simulink model "CtrlrCmptmtT"  (R2022b)
settings: Solver=FixedStepDiscrete  StartTime=0.0  StopTime=10.0  FixedStep=1  RelTol=1e-3
settings Model: Creator=blue.ge  LastModifiedBy=blue.ge
settings DataIOCC: SaveFormat=StructureWithTime
settings OptimizationCC: DefaultParameterBehavior=Inlined  EnhancedBackFolding=on  PassReuseOutputArgsAs=Individual arguments  ZeroExternalMemoryAtStartup=off  ZeroInternalMemoryAtStartup=off  OptimizeBlockOrder=speed  DifferentSizesBufferReuse=on  OptimizationCustomize=off  EfficientTunableParamExpr=on
settings DebuggingCC: ParameterTunabilityLossMsg=error
settings ModelReferenceCC: ModelReferenceNumInstancesAllowed=Single
settings SFSimCC: MATLABDynamicMemAlloc=off
settings RTWCC: Description=AUTOSAR  SystemTargetFile=autosar.tlc  GenCodeOnly=on  TemplateMakefile=ert_default_tmf
settings CodeAppCC: ForceParamTrailComments=on  IgnoreCustomStorageClasses=off  OperatorAnnotations=on  SimulinkDataObjDesc=on  SFDataObjDesc=on  InsertBlockDesc=on
settings STFCustomTargetCC: TargetLangStandard=C99 (ISO)  MultiwordLength=256  ParMdlRefBuildCompliant=on  CompOptLevelCompliant=on  IncludeMdlTerminateFcn=off  CombineOutputUpdateFcns=on  SuppressErrorStatus=on  ERTCustomFileBanners=on  SupportNonFinite=off  SupportComplex=off  SupportContinuousTime=off  SupportNonInlinedSFcns=off  RemoveResetFunc=on  MATLABClassNameForMDSCustomization=Simulink.SoftwareTarget.ERTCustomization  AutosarCompliant=on  UseToolchainInfoCompliant=on  LookupTableObjectStructAxisOrder=2,1,3,4,...  LUTObjectStructOrderEvenSpacing=Size,Table,Breakpoints  SystemTargetFile=autosar.tlc
settings ERTTargetCC: TargetLangStandard=C99 (ISO)  ConcurrentExecutionCompliant=off  IncludeMdlTerminateFcn=off  SuppressErrorStatus=on  SupportNonFinite=off  SupportComplex=off  ModelStepFunctionPrototypeControlCompliant=off  CPPClassGenCompliant=off  AutosarCompliant=on  LookupTableObjectStructAxisOrder=2,1,3,4,...  LUTObjectStructOrderEvenSpacing=Size,Table,Breakpoints  InlinedParameterPlacement=NonHierarchical  ERTCustomFileTemplate=example_file_process.tlc

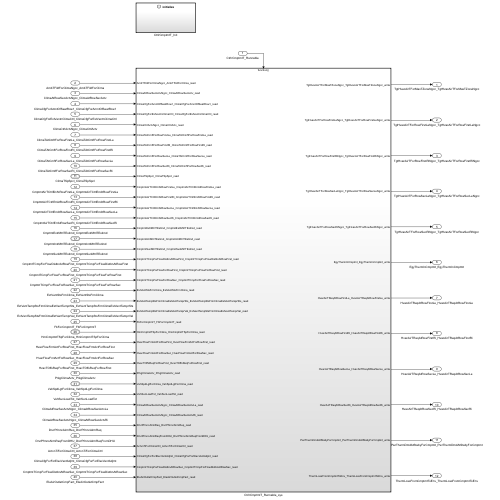
[thumbnail: Simulink's stored preview of the top-level diagram]
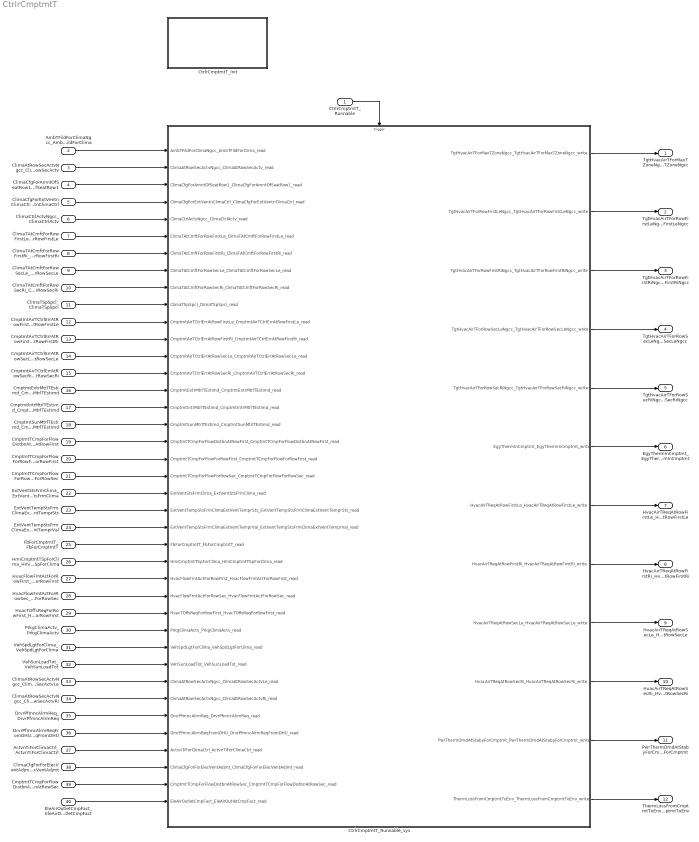
== system CtrlrCmptmtT ==
  CtrlrCmptmtT_Runnable : Inport  SampleTime=1  OutputFunctionCall=on
  AmbTFildForClimaNgcc_AmbTFildForClima : Inport  Port=2  OutDataTypeStr=T30  PortDimensions=1
  ClimaAtRowSecActvNgcc_ClimaAtRowSecActv : Inport  Port=3  OutDataTypeStr=Boolean  PortDimensions=1
  ClimaCfgForAmntOfSeatRow1_ClimaCfgForAmntOfSeatRow1 : Inport  Port=4  OutDataTypeStr=Enum: ClimaCfgForAmntOfSeatRow1  PortDimensions=1
  ClimaCfgForExtVentnClimaCtrl_ClimaCfgForExtVentnClimaCtrl : Inport  Port=5  OutDataTypeStr=Enum: CarCfgForExtVentnClimaCtrl  PortDimensions=1
  ClimaCtrlActvNgcc_ClimaCtrlActv : Inport  Port=6  OutDataTypeStr=Boolean  PortDimensions=1
  ClimaTAtCmftForRowFirstLe_ClimaTAtCmftForRowFirstLe : Inport  Port=7  OutDataTypeStr=T30  PortDimensions=1
  ClimaTAtCmftForRowFirstRi_ClimaTAtCmftForRowFirstRi : Inport  Port=8  OutDataTypeStr=T30  PortDimensions=1
  ClimaTAtCmftForRowSecLe_ClimaTAtCmftForRowSecLe : Inport  Port=9  OutDataTypeStr=T30  PortDimensions=1
  ClimaTAtCmftForRowSecRi_ClimaTAtCmftForRowSecRi : Inport  Port=10  OutDataTypeStr=T30  PortDimensions=1
  ClimaTSpSpcl_ClimaTSpSpcl : Inport  Port=11  OutDataTypeStr=Bus: ClimaTSpSpcl  PortDimensions=1  BusOutputAsStruct=on
  CmptmtAirTCtrlErrAtRowFirstLe_CmptmtAirTCtrlErrAtRowFirstLe : Inport  Port=12  OutDataTypeStr=T30  PortDimensions=1
  CmptmtAirTCtrlErrAtRowFirstRi_CmptmtAirTCtrlErrAtRowFirstRi : Inport  Port=13  OutDataTypeStr=T30  PortDimensions=1
  CmptmtAirTCtrlErrAtRowSecLe_CmptmtAirTCtrlErrAtRowSecLe : Inport  Port=14  OutDataTypeStr=T30  PortDimensions=1
  CmptmtAirTCtrlErrAtRowSecRi_CmptmtAirTCtrlErrAtRowSecRi : Inport  Port=15  OutDataTypeStr=T30  PortDimensions=1
  CmptmtExtrMtrlTEstimd_CmptmtExtrMtrlTEstimd : Inport  Port=16  OutDataTypeStr=T30  PortDimensions=1
  CmptmtIntrMtrlTEstimd_CmptmtIntrMtrlTEstimd : Inport  Port=17  OutDataTypeStr=Bus: MtrlSnsrT  PortDimensions=1  BusOutputAsStruct=on
  CmptmtSunMtrlTEstimd_CmptmtSunMtrlTEstimd : Inport  Port=18  OutDataTypeStr=T30  PortDimensions=1
  CmptmtTCmpForFlowDistbnAtRowFirst_CmptmtTCmpForFlowDistbnAtRowFirst : Inport  Port=19  OutDataTypeStr=Fac30  PortDimensions=1
  CmptmtTCmpForFlowForRowFirst_CmptmtTCmpForFlowForRowFirst : Inport  Port=20  OutDataTypeStr=Fac30  PortDimensions=1
  CmptmtTCmpForFlowForRowSec_CmptmtTCmpForFlowForRowSec : Inport  Port=21  OutDataTypeStr=Fac30  PortDimensions=1
  ExtVentStsFrmClima_ExtVentStsFrmClima : Inport  Port=22  OutDataTypeStr=Enum: OnOff1  PortDimensions=1
  ExtVentTempStsFrmClimaExtVentTemprSts_ExtVentTempStsFrmClimaExtVentTemprSts : Inport  Port=23  OutDataTypeStr=Enum: ExtVentTemprSts  PortDimensions=1
  ExtVentTempStsFrmClimaExtVentTemprVal_ExtVentTempStsFrmClimaExtVentTemprVal : Inport  Port=24  OutDataTypeStr=Temp5  PortDimensions=1
  FbForCmptmtT_FbForCmptmtT : Inport  Port=25  OutDataTypeStr=T30  PortDimensions=1
  HmiCmptmtTSpForClima_HmiCmptmtTSpForClima : Inport  Port=26  OutDataTypeStr=Bus: HmiCmptmtTSp  PortDimensions=1  BusOutputAsStruct=on
  HvacFlowFrntActForRowFirst_HvacFlowFrntActForRowFirst : Inport  Port=27  OutDataTypeStr=AirFlow30  PortDimensions=1
  HvacFlowFrntActForRowSec_HvacFlowFrntActForRowSec : Inport  Port=28  OutDataTypeStr=AirFlow30  PortDimensions=1
  HvacTOffsReqForRowFirst_HvacTOffsReqForRowFirst : Inport  Port=29  OutDataTypeStr=T30  PortDimensions=1
  PrkgClimaActv_PrkgClimaActv : Inport  Port=30  OutDataTypeStr=Enum: PrkgClimaActv  PortDimensions=1
  VehSpdLgtForClima_VehSpdLgtForClima : Inport  Port=31  OutDataTypeStr=Bus: VehSpdLgtForClima  PortDimensions=1  BusOutputAsStruct=on
  VehSunLoadTot_VehSunLoadTot : Inport  Port=32  OutDataTypeStr=SunLoad30  PortDimensions=1
  ClimaAtRowSecActvNgcc_ClimaAtRowSecActvLe : Inport  Port=33  OutDataTypeStr=Boolean  PortDimensions=1
  ClimaAtRowSecActvNgcc_ClimaAtRowSecActvRi : Inport  Port=34  OutDataTypeStr=Boolean  PortDimensions=1
  DrvrPfmncAlrmReq_DrvrPfmncAlrmReq : Inport  Port=35  OutDataTypeStr=Enum: DrvrPfmncWarnReq  PortDimensions=1
  DrvrPfmncAlrmReqFromDHU_DrvrPfmncAlrmReqFromDHU : Inport  Port=36  OutDataTypeStr=Enum: DrvrPfmncWarnReq  PortDimensions=1
  ActvnTiForClimaCtrl_ActvnTiForClimaCtrl : Inport  Port=37  OutDataTypeStr=Ti33  PortDimensions=1
  ClimaCfgForForElecVentAdjmt_ClimaCfgForForElecVentAdjmt : Inport  Port=38  OutDataTypeStr=Boolean  PortDimensions=1
  CmptmtTCmpForFlowDistbnAtRowSec_CmptmtTCmpForFlowDistbnAtRowSec : Inport  Port=39  OutDataTypeStr=Fac30  PortDimensions=1
  EleAirOutletCmpFact_EleAirOutletCmpFact : Inport  Port=40  OutDataTypeStr=VCCFac30  PortDimensions=1
  CtrlrCmptmtT_Init : Subsystem
  CtrlrCmptmtT_Runnable_sys : Subsystem
  TgtHvacAirTForMaxTZoneNgcc_TgtHvacAirTForMaxTZoneNgcc : Outport  OutDataTypeStr=T30  PortDimensions=1
  TgtHvacAirTForRowFirstLeNgcc_TgtHvacAirTForRowFirstLeNgcc : Outport  Port=2  OutMin=-256  OutMax=255.9  OutDataTypeStr=T2  PortDimensions=1
  TgtHvacAirTForRowFirstRiNgcc_TgtHvacAirTForRowFirstRiNgcc : Outport  Port=3  OutMin=-256  OutMax=255.9  OutDataTypeStr=T2  PortDimensions=1
  TgtHvacAirTForRowSecLeNgcc_TgtHvacAirTForRowSecLeNgcc : Outport  Port=4  OutMin=-256  OutMax=255.9  OutDataTypeStr=T2  PortDimensions=1
  TgtHvacAirTForRowSecRiNgcc_TgtHvacAirTForRowSecRiNgcc : Outport  Port=5  OutMin=-256  OutMax=255.9  OutDataTypeStr=T2  PortDimensions=1
  EgyThermInCmptmt_EgyThermInCmptmt : Outport  Port=6  OutDataTypeStr=Egy33  PortDimensions=1
  HvacAirTReqAtRowFirstLe_HvacAirTReqAtRowFirstLe : Outport  Port=7  OutDataTypeStr=T30  PortDimensions=1
  HvacAirTReqAtRowFirstRi_HvacAirTReqAtRowFirstRi : Outport  Port=8  OutDataTypeStr=T30  PortDimensions=1
  HvacAirTReqAtRowSecLe_HvacAirTReqAtRowSecLe : Outport  Port=9  OutDataTypeStr=T30  PortDimensions=1
  HvacAirTReqAtRowSecRi_HvacAirTReqAtRowSecRi : Outport  Port=10  OutDataTypeStr=T30  PortDimensions=1
  PwrThermDmdAtStabyForCmptmt_PwrThermDmdAtStabyForCmptmt : Outport  Port=11  OutDataTypeStr=Pwr33  PortDimensions=1
  ThermLossFromCmptmtToEnv_ThermLossFromCmptmtToEnv : Outport  Port=12  OutDataTypeStr=Pwr33  PortDimensions=1
  wires:
  AmbTFildForClimaNgcc_AmbTFildForClima/1 -> CtrlrCmptmtT_Runnable_sys/1(AmbTFildForClimaNgcc_AmbTFildForClima_read)
  ClimaAtRowSecActvNgcc_ClimaAtRowSecActv/1 -> CtrlrCmptmtT_Runnable_sys/2(ClimaAtRowSecActvNgcc_ClimaAtRowSecActv_read)
  ClimaCfgForAmntOfSeatRow1_ClimaCfgForAmntOfSeatRow1/1 -> CtrlrCmptmtT_Runnable_sys/3(ClimaCfgForAmntOfSeatRow1_ClimaCfgForAmntOfSeatRow1_read)
  ClimaCfgForExtVentnClimaCtrl_ClimaCfgForExtVentnClimaCtrl/1 -> CtrlrCmptmtT_Runnable_sys/4(ClimaCfgForExtVentnClimaCtrl_ClimaCfgForExtVentnClimaCtrl_read)
  ClimaCtrlActvNgcc_ClimaCtrlActv/1 -> CtrlrCmptmtT_Runnable_sys/5(ClimaCtrlActvNgcc_ClimaCtrlActv_read)
  ClimaTAtCmftForRowFirstLe_ClimaTAtCmftForRowFirstLe/1 -> CtrlrCmptmtT_Runnable_sys/6(ClimaTAtCmftForRowFirstLe_ClimaTAtCmftForRowFirstLe_read)
  ClimaTAtCmftForRowFirstRi_ClimaTAtCmftForRowFirstRi/1 -> CtrlrCmptmtT_Runnable_sys/7(ClimaTAtCmftForRowFirstRi_ClimaTAtCmftForRowFirstRi_read)
  ClimaTAtCmftForRowSecLe_ClimaTAtCmftForRowSecLe/1 -> CtrlrCmptmtT_Runnable_sys/8(ClimaTAtCmftForRowSecLe_ClimaTAtCmftForRowSecLe_read)
  ClimaTAtCmftForRowSecRi_ClimaTAtCmftForRowSecRi/1 -> CtrlrCmptmtT_Runnable_sys/9(ClimaTAtCmftForRowSecRi_ClimaTAtCmftForRowSecRi_read)
  ClimaTSpSpcl_ClimaTSpSpcl/1 -> CtrlrCmptmtT_Runnable_sys/10(ClimaTSpSpcl_ClimaTSpSpcl_read)
  CmptmtAirTCtrlErrAtRowFirstLe_CmptmtAirTCtrlErrAtRowFirstLe/1 -> CtrlrCmptmtT_Runnable_sys/11(CmptmtAirTCtrlErrAtRowFirstLe_CmptmtAirTCtrlErrAtRowFirstLe_read)
  CmptmtAirTCtrlErrAtRowFirstRi_CmptmtAirTCtrlErrAtRowFirstRi/1 -> CtrlrCmptmtT_Runnable_sys/12(CmptmtAirTCtrlErrAtRowFirstRi_CmptmtAirTCtrlErrAtRowFirstRi_read)
  CmptmtAirTCtrlErrAtRowSecLe_CmptmtAirTCtrlErrAtRowSecLe/1 -> CtrlrCmptmtT_Runnable_sys/13(CmptmtAirTCtrlErrAtRowSecLe_CmptmtAirTCtrlErrAtRowSecLe_read)
  CmptmtAirTCtrlErrAtRowSecRi_CmptmtAirTCtrlErrAtRowSecRi/1 -> CtrlrCmptmtT_Runnable_sys/14(CmptmtAirTCtrlErrAtRowSecRi_CmptmtAirTCtrlErrAtRowSecRi_read)
  CmptmtExtrMtrlTEstimd_CmptmtExtrMtrlTEstimd/1 -> CtrlrCmptmtT_Runnable_sys/15(CmptmtExtrMtrlTEstimd_CmptmtExtrMtrlTEstimd_read)
  CmptmtIntrMtrlTEstimd_CmptmtIntrMtrlTEstimd/1 -> CtrlrCmptmtT_Runnable_sys/16(CmptmtIntrMtrlTEstimd_CmptmtIntrMtrlTEstimd_read)
  CmptmtSunMtrlTEstimd_CmptmtSunMtrlTEstimd/1 -> CtrlrCmptmtT_Runnable_sys/17(CmptmtSunMtrlTEstimd_CmptmtSunMtrlTEstimd_read)
  CmptmtTCmpForFlowDistbnAtRowFirst_CmptmtTCmpForFlowDistbnAtRowFirst/1 -> CtrlrCmptmtT_Runnable_sys/18(CmptmtTCmpForFlowDistbnAtRowFirst_CmptmtTCmpForFlowDistbnAtRowFirst_read)
  CmptmtTCmpForFlowForRowFirst_CmptmtTCmpForFlowForRowFirst/1 -> CtrlrCmptmtT_Runnable_sys/19(CmptmtTCmpForFlowForRowFirst_CmptmtTCmpForFlowForRowFirst_read)
  CmptmtTCmpForFlowForRowSec_CmptmtTCmpForFlowForRowSec/1 -> CtrlrCmptmtT_Runnable_sys/20(CmptmtTCmpForFlowForRowSec_CmptmtTCmpForFlowForRowSec_read)
  ExtVentStsFrmClima_ExtVentStsFrmClima/1 -> CtrlrCmptmtT_Runnable_sys/21(ExtVentStsFrmClima_ExtVentStsFrmClima_read)
  ExtVentTempStsFrmClimaExtVentTemprSts_ExtVentTempStsFrmClimaExtVentTemprSts/1 -> CtrlrCmptmtT_Runnable_sys/22(ExtVentTempStsFrmClimaExtVentTemprSts_ExtVentTempStsFrmClimaExtVentTemprSts_read)
  ExtVentTempStsFrmClimaExtVentTemprVal_ExtVentTempStsFrmClimaExtVentTemprVal/1 -> CtrlrCmptmtT_Runnable_sys/23(ExtVentTempStsFrmClimaExtVentTemprVal_ExtVentTempStsFrmClimaExtVentTemprVal_read)
  FbForCmptmtT_FbForCmptmtT/1 -> CtrlrCmptmtT_Runnable_sys/24(FbForCmptmtT_FbForCmptmtT_read)
  HmiCmptmtTSpForClima_HmiCmptmtTSpForClima/1 -> CtrlrCmptmtT_Runnable_sys/25(HmiCmptmtTSpForClima_HmiCmptmtTSpForClima_read)
  HvacFlowFrntActForRowFirst_HvacFlowFrntActForRowFirst/1 -> CtrlrCmptmtT_Runnable_sys/26(HvacFlowFrntActForRowFirst_HvacFlowFrntActForRowFirst_read)
  HvacFlowFrntActForRowSec_HvacFlowFrntActForRowSec/1 -> CtrlrCmptmtT_Runnable_sys/27(HvacFlowFrntActForRowSec_HvacFlowFrntActForRowSec_read)
  HvacTOffsReqForRowFirst_HvacTOffsReqForRowFirst/1 -> CtrlrCmptmtT_Runnable_sys/28(HvacTOffsReqForRowFirst_HvacTOffsReqForRowFirst_read)
  PrkgClimaActv_PrkgClimaActv/1 -> CtrlrCmptmtT_Runnable_sys/29(PrkgClimaActv_PrkgClimaActv_read)
  VehSpdLgtForClima_VehSpdLgtForClima/1 -> CtrlrCmptmtT_Runnable_sys/30(VehSpdLgtForClima_VehSpdLgtForClima_read)
  VehSunLoadTot_VehSunLoadTot/1 -> CtrlrCmptmtT_Runnable_sys/31(VehSunLoadTot_VehSunLoadTot_read)
  ClimaAtRowSecActvNgcc_ClimaAtRowSecActvLe/1 -> CtrlrCmptmtT_Runnable_sys/32(ClimaAtRowSecActvNgcc_ClimaAtRowSecActvLe_read)
  ClimaAtRowSecActvNgcc_ClimaAtRowSecActvRi/1 -> CtrlrCmptmtT_Runnable_sys/33(ClimaAtRowSecActvNgcc_ClimaAtRowSecActvRi_read)
  DrvrPfmncAlrmReq_DrvrPfmncAlrmReq/1 -> CtrlrCmptmtT_Runnable_sys/34(DrvrPfmncAlrmReq_DrvrPfmncAlrmReq_read)
  DrvrPfmncAlrmReqFromDHU_DrvrPfmncAlrmReqFromDHU/1 -> CtrlrCmptmtT_Runnable_sys/35(DrvrPfmncAlrmReqFromDHU_DrvrPfmncAlrmReqFromDHU_read)
  ActvnTiForClimaCtrl_ActvnTiForClimaCtrl/1 -> CtrlrCmptmtT_Runnable_sys/36(ActvnTiForClimaCtrl_ActvnTiForClimaCtrl_read)
  ClimaCfgForForElecVentAdjmt_ClimaCfgForForElecVentAdjmt/1 -> CtrlrCmptmtT_Runnable_sys/37(ClimaCfgForForElecVentAdjmt_ClimaCfgForForElecVentAdjmt_read)
  CmptmtTCmpForFlowDistbnAtRowSec_CmptmtTCmpForFlowDistbnAtRowSec/1 -> CtrlrCmptmtT_Runnable_sys/38(CmptmtTCmpForFlowDistbnAtRowSec_CmptmtTCmpForFlowDistbnAtRowSec_read)
  EleAirOutletCmpFact_EleAirOutletCmpFact/1 -> CtrlrCmptmtT_Runnable_sys/39(EleAirOutletCmpFact_EleAirOutletCmpFact_read)
  CtrlrCmptmtT_Runnable_sys/1(TgtHvacAirTForMaxTZoneNgcc_TgtHvacAirTForMaxTZoneNgcc_write) -> TgtHvacAirTForMaxTZoneNgcc_TgtHvacAirTForMaxTZoneNgcc/1
  CtrlrCmptmtT_Runnable_sys/2(TgtHvacAirTForRowFirstLeNgcc_TgtHvacAirTForRowFirstLeNgcc_write) -> TgtHvacAirTForRowFirstLeNgcc_TgtHvacAirTForRowFirstLeNgcc/1
  CtrlrCmptmtT_Runnable_sys/3(TgtHvacAirTForRowFirstRiNgcc_TgtHvacAirTForRowFirstRiNgcc_write) -> TgtHvacAirTForRowFirstRiNgcc_TgtHvacAirTForRowFirstRiNgcc/1
  CtrlrCmptmtT_Runnable_sys/4(TgtHvacAirTForRowSecLeNgcc_TgtHvacAirTForRowSecLeNgcc_write) -> TgtHvacAirTForRowSecLeNgcc_TgtHvacAirTForRowSecLeNgcc/1
  CtrlrCmptmtT_Runnable_sys/5(TgtHvacAirTForRowSecRiNgcc_TgtHvacAirTForRowSecRiNgcc_write) -> TgtHvacAirTForRowSecRiNgcc_TgtHvacAirTForRowSecRiNgcc/1
  CtrlrCmptmtT_Runnable_sys/6(EgyThermInCmptmt_EgyThermInCmptmt_write) -> EgyThermInCmptmt_EgyThermInCmptmt/1
  CtrlrCmptmtT_Runnable_sys/7(HvacAirTReqAtRowFirstLe_HvacAirTReqAtRowFirstLe_write) -> HvacAirTReqAtRowFirstLe_HvacAirTReqAtRowFirstLe/1
  CtrlrCmptmtT_Runnable_sys/8(HvacAirTReqAtRowFirstRi_HvacAirTReqAtRowFirstRi_write) -> HvacAirTReqAtRowFirstRi_HvacAirTReqAtRowFirstRi/1
  CtrlrCmptmtT_Runnable_sys/9(HvacAirTReqAtRowSecLe_HvacAirTReqAtRowSecLe_write) -> HvacAirTReqAtRowSecLe_HvacAirTReqAtRowSecLe/1
  CtrlrCmptmtT_Runnable_sys/10(HvacAirTReqAtRowSecRi_HvacAirTReqAtRowSecRi_write) -> HvacAirTReqAtRowSecRi_HvacAirTReqAtRowSecRi/1
  CtrlrCmptmtT_Runnable_sys/11(PwrThermDmdAtStabyForCmptmt_PwrThermDmdAtStabyForCmptmt_write) -> PwrThermDmdAtStabyForCmptmt_PwrThermDmdAtStabyForCmptmt/1
  CtrlrCmptmtT_Runnable_sys/12(ThermLossFromCmptmtToEnv_ThermLossFromCmptmtToEnv_write) -> ThermLossFromCmptmtToEnv_ThermLossFromCmptmtToEnv/1
  CtrlrCmptmtT_Runnable/1 -> CtrlrCmptmtT_Runnable_sys/Trigger

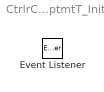
== system CtrlrCmptmtT/CtrlrCmptmtT_Init ==
  Event Listener : EventListener  EventName=reset

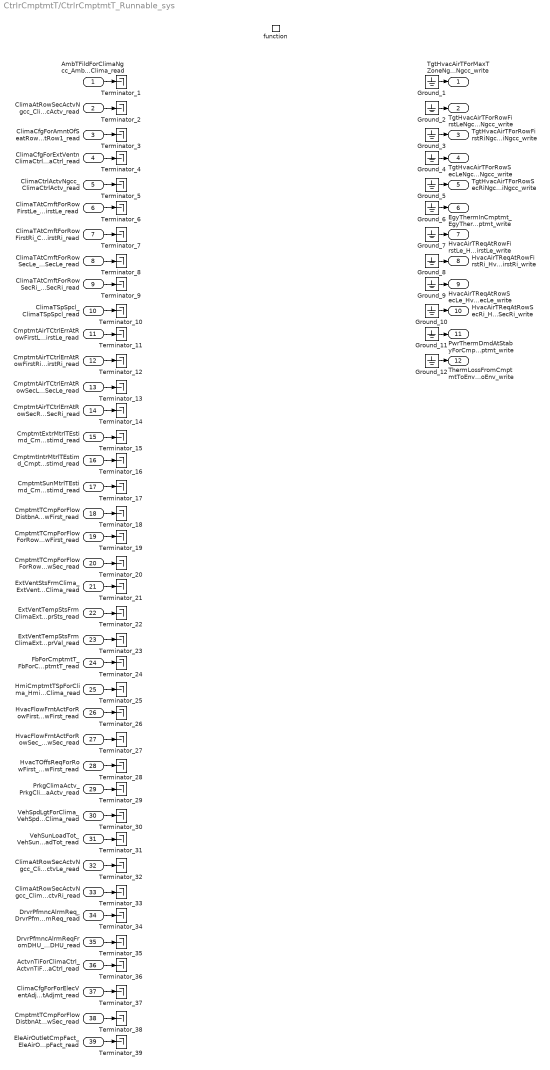
== system CtrlrCmptmtT/CtrlrCmptmtT_Runnable_sys ==
  AmbTFildForClimaNgcc_AmbTFildForClima_read : Inport
  ClimaAtRowSecActvNgcc_ClimaAtRowSecActv_read : Inport  Port=2
  ClimaCfgForAmntOfSeatRow1_ClimaCfgForAmntOfSeatRow1_read : Inport  Port=3
  ClimaCfgForExtVentnClimaCtrl_ClimaCfgForExtVentnClimaCtrl_read : Inport  Port=4
  ClimaCtrlActvNgcc_ClimaCtrlActv_read : Inport  Port=5
  ClimaTAtCmftForRowFirstLe_ClimaTAtCmftForRowFirstLe_read : Inport  Port=6
  ClimaTAtCmftForRowFirstRi_ClimaTAtCmftForRowFirstRi_read : Inport  Port=7
  ClimaTAtCmftForRowSecLe_ClimaTAtCmftForRowSecLe_read : Inport  Port=8
  ClimaTAtCmftForRowSecRi_ClimaTAtCmftForRowSecRi_read : Inport  Port=9
  ClimaTSpSpcl_ClimaTSpSpcl_read : Inport  Port=10
  CmptmtAirTCtrlErrAtRowFirstLe_CmptmtAirTCtrlErrAtRowFirstLe_read : Inport  Port=11
  CmptmtAirTCtrlErrAtRowFirstRi_CmptmtAirTCtrlErrAtRowFirstRi_read : Inport  Port=12
  CmptmtAirTCtrlErrAtRowSecLe_CmptmtAirTCtrlErrAtRowSecLe_read : Inport  Port=13
  CmptmtAirTCtrlErrAtRowSecRi_CmptmtAirTCtrlErrAtRowSecRi_read : Inport  Port=14
  CmptmtExtrMtrlTEstimd_CmptmtExtrMtrlTEstimd_read : Inport  Port=15
  CmptmtIntrMtrlTEstimd_CmptmtIntrMtrlTEstimd_read : Inport  Port=16
  CmptmtSunMtrlTEstimd_CmptmtSunMtrlTEstimd_read : Inport  Port=17
  CmptmtTCmpForFlowDistbnAtRowFirst_CmptmtTCmpForFlowDistbnAtRowFirst_read : Inport  Port=18
  CmptmtTCmpForFlowForRowFirst_CmptmtTCmpForFlowForRowFirst_read : Inport  Port=19
  CmptmtTCmpForFlowForRowSec_CmptmtTCmpForFlowForRowSec_read : Inport  Port=20
  ExtVentStsFrmClima_ExtVentStsFrmClima_read : Inport  Port=21
  ExtVentTempStsFrmClimaExtVentTemprSts_ExtVentTempStsFrmClimaExtVentTemprSts_read : Inport  Port=22
  ExtVentTempStsFrmClimaExtVentTemprVal_ExtVentTempStsFrmClimaExtVentTemprVal_read : Inport  Port=23
  FbForCmptmtT_FbForCmptmtT_read : Inport  Port=24
  HmiCmptmtTSpForClima_HmiCmptmtTSpForClima_read : Inport  Port=25
  HvacFlowFrntActForRowFirst_HvacFlowFrntActForRowFirst_read : Inport  Port=26
  HvacFlowFrntActForRowSec_HvacFlowFrntActForRowSec_read : Inport  Port=27
  HvacTOffsReqForRowFirst_HvacTOffsReqForRowFirst_read : Inport  Port=28
  PrkgClimaActv_PrkgClimaActv_read : Inport  Port=29
  VehSpdLgtForClima_VehSpdLgtForClima_read : Inport  Port=30
  VehSunLoadTot_VehSunLoadTot_read : Inport  Port=31
  ClimaAtRowSecActvNgcc_ClimaAtRowSecActvLe_read : Inport  Port=32
  ClimaAtRowSecActvNgcc_ClimaAtRowSecActvRi_read : Inport  Port=33
  DrvrPfmncAlrmReq_DrvrPfmncAlrmReq_read : Inport  Port=34
  DrvrPfmncAlrmReqFromDHU_DrvrPfmncAlrmReqFromDHU_read : Inport  Port=35
  ActvnTiForClimaCtrl_ActvnTiForClimaCtrl_read : Inport  Port=36
  ClimaCfgForForElecVentAdjmt_ClimaCfgForForElecVentAdjmt_read : Inport  Port=37
  CmptmtTCmpForFlowDistbnAtRowSec_CmptmtTCmpForFlowDistbnAtRowSec_read : Inport  Port=38
  EleAirOutletCmpFact_EleAirOutletCmpFact_read : Inport  Port=39
  function : TriggerPort  TriggerType=function-call  VariantControl=(inherit)  SampleTimeType=periodic  SampleTime=-1
  Ground_{1..12} (12 alike) : Ground
  Terminator_{1..39} (39 alike) : Terminator
  TgtHvacAirTForMaxTZoneNgcc_TgtHvacAirTForMaxTZoneNgcc_write : Outport
      settings: EnsureOutportIsVirtual=on
  TgtHvacAirTForRowFirstLeNgcc_TgtHvacAirTForRowFirstLeNgcc_write : Outport  Port=2
      settings: EnsureOutportIsVirtual=on
  TgtHvacAirTForRowFirstRiNgcc_TgtHvacAirTForRowFirstRiNgcc_write : Outport  Port=3
      settings: EnsureOutportIsVirtual=on
  TgtHvacAirTForRowSecLeNgcc_TgtHvacAirTForRowSecLeNgcc_write : Outport  Port=4
      settings: EnsureOutportIsVirtual=on
  TgtHvacAirTForRowSecRiNgcc_TgtHvacAirTForRowSecRiNgcc_write : Outport  Port=5
      settings: EnsureOutportIsVirtual=on
  EgyThermInCmptmt_EgyThermInCmptmt_write : Outport  Port=6
      settings: EnsureOutportIsVirtual=on
  HvacAirTReqAtRowFirstLe_HvacAirTReqAtRowFirstLe_write : Outport  Port=7
      settings: EnsureOutportIsVirtual=on
  HvacAirTReqAtRowFirstRi_HvacAirTReqAtRowFirstRi_write : Outport  Port=8
      settings: EnsureOutportIsVirtual=on
  HvacAirTReqAtRowSecLe_HvacAirTReqAtRowSecLe_write : Outport  Port=9
      settings: EnsureOutportIsVirtual=on
  HvacAirTReqAtRowSecRi_HvacAirTReqAtRowSecRi_write : Outport  Port=10
      settings: EnsureOutportIsVirtual=on
  PwrThermDmdAtStabyForCmptmt_PwrThermDmdAtStabyForCmptmt_write : Outport  Port=11
      settings: EnsureOutportIsVirtual=on
  ThermLossFromCmptmtToEnv_ThermLossFromCmptmtToEnv_write : Outport  Port=12
      settings: EnsureOutportIsVirtual=on
  wires:
  AmbTFildForClimaNgcc_AmbTFildForClima_read/1 -> Terminator_1/1
  ClimaAtRowSecActvNgcc_ClimaAtRowSecActv_read/1 -> Terminator_2/1
  ClimaCfgForAmntOfSeatRow1_ClimaCfgForAmntOfSeatRow1_read/1 -> Terminator_3/1
  ClimaCfgForExtVentnClimaCtrl_ClimaCfgForExtVentnClimaCtrl_read/1 -> Terminator_4/1
  ClimaCtrlActvNgcc_ClimaCtrlActv_read/1 -> Terminator_5/1
  ClimaTAtCmftForRowFirstLe_ClimaTAtCmftForRowFirstLe_read/1 -> Terminator_6/1
  ClimaTAtCmftForRowFirstRi_ClimaTAtCmftForRowFirstRi_read/1 -> Terminator_7/1
  ClimaTAtCmftForRowSecLe_ClimaTAtCmftForRowSecLe_read/1 -> Terminator_8/1
  ClimaTAtCmftForRowSecRi_ClimaTAtCmftForRowSecRi_read/1 -> Terminator_9/1
  ClimaTSpSpcl_ClimaTSpSpcl_read/1 -> Terminator_10/1
  CmptmtAirTCtrlErrAtRowFirstLe_CmptmtAirTCtrlErrAtRowFirstLe_read/1 -> Terminator_11/1
  CmptmtAirTCtrlErrAtRowFirstRi_CmptmtAirTCtrlErrAtRowFirstRi_read/1 -> Terminator_12/1
  CmptmtAirTCtrlErrAtRowSecLe_CmptmtAirTCtrlErrAtRowSecLe_read/1 -> Terminator_13/1
  CmptmtAirTCtrlErrAtRowSecRi_CmptmtAirTCtrlErrAtRowSecRi_read/1 -> Terminator_14/1
  CmptmtExtrMtrlTEstimd_CmptmtExtrMtrlTEstimd_read/1 -> Terminator_15/1
  CmptmtIntrMtrlTEstimd_CmptmtIntrMtrlTEstimd_read/1 -> Terminator_16/1
  CmptmtSunMtrlTEstimd_CmptmtSunMtrlTEstimd_read/1 -> Terminator_17/1
  CmptmtTCmpForFlowDistbnAtRowFirst_CmptmtTCmpForFlowDistbnAtRowFirst_read/1 -> Terminator_18/1
  CmptmtTCmpForFlowForRowFirst_CmptmtTCmpForFlowForRowFirst_read/1 -> Terminator_19/1
  CmptmtTCmpForFlowForRowSec_CmptmtTCmpForFlowForRowSec_read/1 -> Terminator_20/1
  ExtVentStsFrmClima_ExtVentStsFrmClima_read/1 -> Terminator_21/1
  ExtVentTempStsFrmClimaExtVentTemprSts_ExtVentTempStsFrmClimaExtVentTemprSts_read/1 -> Terminator_22/1
  ExtVentTempStsFrmClimaExtVentTemprVal_ExtVentTempStsFrmClimaExtVentTemprVal_read/1 -> Terminator_23/1
  FbForCmptmtT_FbForCmptmtT_read/1 -> Terminator_24/1
  HmiCmptmtTSpForClima_HmiCmptmtTSpForClima_read/1 -> Terminator_25/1
  HvacFlowFrntActForRowFirst_HvacFlowFrntActForRowFirst_read/1 -> Terminator_26/1
  HvacFlowFrntActForRowSec_HvacFlowFrntActForRowSec_read/1 -> Terminator_27/1
  HvacTOffsReqForRowFirst_HvacTOffsReqForRowFirst_read/1 -> Terminator_28/1
  PrkgClimaActv_PrkgClimaActv_read/1 -> Terminator_29/1
  VehSpdLgtForClima_VehSpdLgtForClima_read/1 -> Terminator_30/1
  VehSunLoadTot_VehSunLoadTot_read/1 -> Terminator_31/1
  ClimaAtRowSecActvNgcc_ClimaAtRowSecActvLe_read/1 -> Terminator_32/1
  ClimaAtRowSecActvNgcc_ClimaAtRowSecActvRi_read/1 -> Terminator_33/1
  DrvrPfmncAlrmReq_DrvrPfmncAlrmReq_read/1 -> Terminator_34/1
  DrvrPfmncAlrmReqFromDHU_DrvrPfmncAlrmReqFromDHU_read/1 -> Terminator_35/1
  ActvnTiForClimaCtrl_ActvnTiForClimaCtrl_read/1 -> Terminator_36/1
  ClimaCfgForForElecVentAdjmt_ClimaCfgForForElecVentAdjmt_read/1 -> Terminator_37/1
  CmptmtTCmpForFlowDistbnAtRowSec_CmptmtTCmpForFlowDistbnAtRowSec_read/1 -> Terminator_38/1
  EleAirOutletCmpFact_EleAirOutletCmpFact_read/1 -> Terminator_39/1
  Ground_1/1 -> TgtHvacAirTForMaxTZoneNgcc_TgtHvacAirTForMaxTZoneNgcc_write/1
  Ground_2/1 -> TgtHvacAirTForRowFirstLeNgcc_TgtHvacAirTForRowFirstLeNgcc_write/1
  Ground_3/1 -> TgtHvacAirTForRowFirstRiNgcc_TgtHvacAirTForRowFirstRiNgcc_write/1
  Ground_4/1 -> TgtHvacAirTForRowSecLeNgcc_TgtHvacAirTForRowSecLeNgcc_write/1
  Ground_5/1 -> TgtHvacAirTForRowSecRiNgcc_TgtHvacAirTForRowSecRiNgcc_write/1
  Ground_6/1 -> EgyThermInCmptmt_EgyThermInCmptmt_write/1
  Ground_7/1 -> HvacAirTReqAtRowFirstLe_HvacAirTReqAtRowFirstLe_write/1
  Ground_8/1 -> HvacAirTReqAtRowFirstRi_HvacAirTReqAtRowFirstRi_write/1
  Ground_9/1 -> HvacAirTReqAtRowSecLe_HvacAirTReqAtRowSecLe_write/1
  Ground_10/1 -> HvacAirTReqAtRowSecRi_HvacAirTReqAtRowSecRi_write/1
  Ground_11/1 -> PwrThermDmdAtStabyForCmptmt_PwrThermDmdAtStabyForCmptmt_write/1
  Ground_12/1 -> ThermLossFromCmptmtToEnv_ThermLossFromCmptmtToEnv_write/1
